simulink model "non_linear_MBS_PF_final"  (R2021b)
settings: Solver=ode15s  StartTime=0  StopTime=SimTime  FixedStep=.00001  RelTol=1e-3
values from neighbouring files (symbols this model uses):
  A_ratio = 1.41    [non_linear_param_MBS_PF_final.m]    (also in non_linear_param_final.m)
  Annulus_area = Bore_area-Rod_area    (= 2356.19449019)    [non_linear_param_MBS_PF_final.m]    (also in non_linear_param_final.m)
  beta_F = 12000e5    (= 1200000000)    [non_linear_param_MBS_PF_final.m]    (also in non_linear_param_final.m)
  Bore_area = pi/4*Bore_diameter^2    (= 3318.30724035)    [non_linear_param_MBS_PF_final.m]    (also in non_linear_param_final.m)
  cyl_c_end = 9e8    (= 900000000)    [non_linear_param_MBS_PF_final.m]    (also in non_linear_param_final.m)
  cyl_c_visc = 1500    [non_linear_param_MBS_PF_final.m]    (also in non_linear_param_final.m)
  cyl_CH_stat = 1/50    (= 0.02)    [non_linear_param_MBS_PF_final.m]    (also in non_linear_param_final.m)
  cyl_F_coul = 75    [non_linear_param_MBS_PF_final.m]    (also in non_linear_param_final.m)
  cyl_FH_stat = 500    [non_linear_param_MBS_PF_final.m]    (also in non_linear_param_final.m)
  cyl_gamma = 250    [non_linear_param_MBS_PF_final.m]    (also in non_linear_param_final.m)
  cyl_k_end = 6e9    (= 6000000000)    [non_linear_param_MBS_PF_final.m]    (also in non_linear_param_final.m)
  cyl_KLi = 0    [non_linear_param_MBS_PF_final.m]    (also in non_linear_param_final.m)
  d_eff_long = sqrt(2*J_sec_long*k_eff_long)    [non_linear_param_MBS_PF_final.m]    (also in non_linear_param_final.m)
  d_eff_short = sqrt(2*J_sec_short*k_eff_short)    [non_linear_param_MBS_PF_final.m]    (also in non_linear_param_final.m)
  DB = 0    [non_linear_param_MBS_PF_final.m]    (also in non_linear_param_final.m = -0.114)
  DR = 1    [non_linear_param_MBS_PF_final.m]    (also in non_linear_param_final.m)
  dr_dcv = 0.7    [non_linear_param_MBS_PF_final.m]    (also in non_linear_param_final.m)
  Eff_Bulk_On = 1    [non_linear_param_MBS_PF_final.m]    (also in non_linear_param_final.m)
  eta_air = 0.7e-2    (= 0.007)    [non_linear_param_MBS_PF_final.m]    (also in non_linear_param_final.m)
  k_cv = 15    [non_linear_param_MBS_PF_final.m]    (also in non_linear_param_final.m)
  k_DCV_PA = 7.85    [non_linear_param_MBS_PF_final.m]    (also in non_linear_param_final.m)
  k_DCV_PB = 7.55    [non_linear_param_MBS_PF_final.m]    (also in non_linear_param_final.m)
  k_DCV_T = 7.85    [non_linear_param_MBS_PF_final.m]    (also in non_linear_param_final.m)
  k_eff_long = E*I/I_sec_long    (= 3050381.94041)    [non_linear_param_MBS_PF_final.m]    (also in non_linear_param_final.m)
  k_eff_short = E*I/I_sec_short    (= 13451432.5427)    [non_linear_param_MBS_PF_final.m]    (also in non_linear_param_final.m)
  k_ocv = 15    [non_linear_param_MBS_PF_final.m]    (also in non_linear_param_final.m)
  K_oil = 1.5e4*0+12000    (= 12000)    [non_linear_param_MBS_PF_final.m]    (also in non_linear_param_final.m)
  k_tanh = 0.005    [non_linear_param_MBS_PF_final.m]    (also in non_linear_param_final.m)
  kappa_air = 1.4    [non_linear_param_MBS_PF_final.m]    (also in non_linear_param_final.m)
  kHP = C*kF_pf_Up    (= 2.90878e-12)    [non_linear_param_MBS_PF_final.m]
  kI = (0.54*ku)/Ti    (= 0.048)    [non_linear_param_MBS_PF_final.m]
  kP = 0.45*ku    (= 0.018)    [non_linear_param_MBS_PF_final.m]
  kq_xS_PB = 4.8946e-04    (= 0.00048946)    [non_linear_param_MBS_PF_final.m]
  KR = 1    [non_linear_param_MBS_PF_final.m]    (also in non_linear_param_final.m)
  mu_ocv = 10    [non_linear_param_MBS_PF_final.m]    (also in non_linear_param_final.m = 3)
  muC = (Bore_diameter^2)/(Bore_diameter^2-Rod_diameter^2)    (= 1.40833333333)    [non_linear_param_MBS_PF_final.m]
  OL_pa = -0.114    [non_linear_param_MBS_PF_final.m]    (also in non_linear_param_final.m)
  OL_pb = 0.114    [non_linear_param_MBS_PF_final.m]    (also in non_linear_param_final.m)
  p0_A = 62.79    [non_linear_param_MBS_PF_final.m]    (also in non_linear_param_final.m)
  p0_A1 = 1.08    [non_linear_param_MBS_PF_final.m]    (also in non_linear_param_final.m)
  p0_B = 1.213    [non_linear_param_MBS_PF_final.m]    (also in non_linear_param_final.m)
  p_cv_fo = 1    [non_linear_param_MBS_PF_final.m]    (also in non_linear_param_final.m)
  p_cv_set = 2.8    [non_linear_param_MBS_PF_final.m]    (also in non_linear_param_final.m)
  p_ocv_fo = 350    [non_linear_param_MBS_PF_final.m]    (also in non_linear_param_final.m)
  p_ocv_set = 196    [non_linear_param_MBS_PF_final.m]    (also in non_linear_param_final.m = 150)
  p_pc_set = 7    [non_linear_param_MBS_PF_final.m]    (also in non_linear_param_final.m)
  p_R = 1.1    [non_linear_param_MBS_PF_final.m]    (also in non_linear_param_final.m)
  p_S = 180    [non_linear_param_MBS_PF_final.m]    (also in non_linear_param_final.m)
  patm = 1.013e5    (= 101300)    [non_linear_param_MBS_PF_final.m]    (also in non_linear_param_final.m)
  Stroke = 500    [non_linear_param_MBS_PF_final.m]    (also in non_linear_param_final.m)
  t0 = 3    [non_linear_param_MBS_PF_final.m]    (also in non_linear_param_final.m = 1)
  tau_ocv = 0.0889*0+0.001    (= 0.001)    [non_linear_param_MBS_PF_final.m]    (also in non_linear_param_final.m)
  tau_pc = tau_ocv    (= 0.001)    [non_linear_param_MBS_PF_final.m]    (also in non_linear_param_final.m)
  tHP = tF_pf_Up/(wn_pf)    (= 0.114613180516)    [non_linear_param_MBS_PF_final.m]
  tStepDown = 4.7*0+7.5    (= 7.5)    [non_linear_param_MBS_PF_final.m]    (also in non_linear_param_final.m = 4.6)
  tStepUp = 10.0    (= 10)    [non_linear_param_MBS_PF_final.m]    (also in non_linear_param_final.m = 6.2)
  tWait = 5.0    (= 5)    [non_linear_param_MBS_PF_final.m]    (also in non_linear_param_final.m = 8)
  UL_at = -0.05    [non_linear_param_MBS_PF_final.m]    (also in non_linear_param_final.m)
  UL_bt = 0.05    [non_linear_param_MBS_PF_final.m]    (also in non_linear_param_final.m)
  Up = 1    [non_linear_param_MBS_PF_final.m]    (also in non_linear_param_final.m)
  uS_sp = -0.4    [non_linear_param_MBS_PF_final.m]    (also in non_linear_param_final.m = -0.55)
  V_CH_A = 1.0e-03    (= 0.001)    [non_linear_param_MBS_PF_final.m]    (also in non_linear_param_final.m)
  V_CH_A1 = 0.1e-03    (= 0.0001)    [non_linear_param_MBS_PF_final.m]    (also in non_linear_param_final.m)
  V_CH_B = 1.25e-03    (= 0.00125)    [non_linear_param_MBS_PF_final.m]    (also in non_linear_param_final.m)
  wn_dcv = 30    [non_linear_param_MBS_PF_final.m]    (also in non_linear_param_final.m)
undefined symbols (not defined in the file or in its folder): sigbuilder_block, smiData, uS0, X, Y, Z
libraries: sm_lib x189, nesl_utility x4, eeGeneralControl x2
(part 6 of 6)

== system non_linear_MBS_PF_final/Test/Subsystem2/Base/Base_profil_base_support_1_RIGID ==
  ReferenceFrame : sm_lib/Frames and Transforms/Reference Frame
  Solid : sm_lib/Body Elements/File Solid  ExtGeomFileName=Base_profil_base_support_Default_sldprt.STEP  UnitType=FromFile  ExtGeomFileUnits=m  StepReaderType=HEX  ExportConvexHullGeometry=off  InertiaType=Custom  Mass=smiData.Solid(3).mass  MassUnits=kg  CenterOfMass=smiData.Solid(3).CoM  CenterOfMassUnits=mm  MomentsOfInertia=smiData.Solid(3).MoI  MomentsOfInertiaUnits=kg*mm^2  ProductsOfInertia=smiData.Solid(3).PoI  ProductsOfInertiaUnits=kg*mm^2  BasedOnType=Density  Density=1000  DensityUnits=kg/m^3  DoExposeReferenceFrame=on  SerializedFrames=<Frames/>  GraphicType=FromGeometry  MarkerShape=Sphere  MarkerSize=10  GraphicVisPropType=SimpleVisualProperties  GraphicDiffuseColor=smiData.Solid(3).color  GraphicSpecularColor=[0.5 0.5 0.5 1.0]  GraphicAmbientColor=[0.15 0.15 0.15 1.0]  GraphicEmissiveColor=[0.0 0.0 0.0 1.0]  GraphicShininess=75  GraphicOpacity=smiData.Solid(3).opacity
  F : PMIOPort  Side=Left
  connections:
  Solid/RConn1 -- ReferenceFrame/RConn1 -- F/RConn1

== system non_linear_MBS_PF_final/Test/Subsystem2/Base/Base_profil_base_support_2_1_RIGID ==
  ReferenceFrame : sm_lib/Frames and Transforms/Reference Frame
  Solid : sm_lib/Body Elements/File Solid  ExtGeomFileName=Base_profil_base_support_2_Default_sldprt.STEP  UnitType=FromFile  ExtGeomFileUnits=m  StepReaderType=HEX  ExportConvexHullGeometry=off  InertiaType=Custom  Mass=smiData.Solid(5).mass  MassUnits=kg  CenterOfMass=smiData.Solid(5).CoM  CenterOfMassUnits=mm  MomentsOfInertia=smiData.Solid(5).MoI  MomentsOfInertiaUnits=kg*mm^2  ProductsOfInertia=smiData.Solid(5).PoI  ProductsOfInertiaUnits=kg*mm^2  BasedOnType=Density  Density=1000  DensityUnits=kg/m^3  DoExposeReferenceFrame=on  SerializedFrames=<Frames/>  GraphicType=FromGeometry  MarkerShape=Sphere  MarkerSize=10  GraphicVisPropType=SimpleVisualProperties  GraphicDiffuseColor=smiData.Solid(5).color  GraphicSpecularColor=[0.5 0.5 0.5 1.0]  GraphicAmbientColor=[0.15 0.15 0.15 1.0]  GraphicEmissiveColor=[0.0 0.0 0.0 1.0]  GraphicShininess=75  GraphicOpacity=smiData.Solid(5).opacity
  F : PMIOPort  Side=Left
  connections:
  Solid/RConn1 -- ReferenceFrame/RConn1 -- F/RConn1

== system non_linear_MBS_PF_final/Test/Subsystem2/Base/Base_profil_cyl_mount1_1_RIGID ==
  ReferenceFrame : sm_lib/Frames and Transforms/Reference Frame
  Solid : sm_lib/Body Elements/File Solid  ExtGeomFileName=Base_profil_cyl_mount1_Default_sldprt.STEP  UnitType=FromFile  ExtGeomFileUnits=m  StepReaderType=HEX  ExportConvexHullGeometry=off  InertiaType=Custom  Mass=smiData.Solid(11).mass  MassUnits=kg  CenterOfMass=smiData.Solid(11).CoM  CenterOfMassUnits=mm  MomentsOfInertia=smiData.Solid(11).MoI  MomentsOfInertiaUnits=kg*mm^2  ProductsOfInertia=smiData.Solid(11).PoI  ProductsOfInertiaUnits=kg*mm^2  BasedOnType=Density  Density=1000  DensityUnits=kg/m^3  DoExposeReferenceFrame=on  SerializedFrames=<Frames/>  GraphicType=FromGeometry  MarkerShape=Sphere  MarkerSize=10  GraphicVisPropType=SimpleVisualProperties  GraphicDiffuseColor=smiData.Solid(11).color  GraphicSpecularColor=[0.5 0.5 0.5 1.0]  GraphicAmbientColor=[0.15 0.15 0.15 1.0]  GraphicEmissiveColor=[0.0 0.0 0.0 1.0]  GraphicShininess=75  GraphicOpacity=smiData.Solid(11).opacity
  F : PMIOPort  Side=Left
  connections:
  Solid/RConn1 -- ReferenceFrame/RConn1 -- F/RConn1

== system non_linear_MBS_PF_final/Test/Subsystem2/Base/Base_profil_cyl_mount_support_sideways_1_RIGID ==
  ReferenceFrame : sm_lib/Frames and Transforms/Reference Frame
  Solid : sm_lib/Body Elements/File Solid  ExtGeomFileName=Base_profil_cyl_mount_support_sideways_Default_sldprt.STEP  UnitType=FromFile  ExtGeomFileUnits=m  StepReaderType=HEX  ExportConvexHullGeometry=off  InertiaType=Custom  Mass=smiData.Solid(2).mass  MassUnits=kg  CenterOfMass=smiData.Solid(2).CoM  CenterOfMassUnits=mm  MomentsOfInertia=smiData.Solid(2).MoI  MomentsOfInertiaUnits=kg*mm^2  ProductsOfInertia=smiData.Solid(2).PoI  ProductsOfInertiaUnits=kg*mm^2  BasedOnType=Density  Density=1000  DensityUnits=kg/m^3  DoExposeReferenceFrame=on  SerializedFrames=<Frames/>  GraphicType=FromGeometry  MarkerShape=Sphere  MarkerSize=10  GraphicVisPropType=SimpleVisualProperties  GraphicDiffuseColor=smiData.Solid(2).color  GraphicSpecularColor=[0.5 0.5 0.5 1.0]  GraphicAmbientColor=[0.15 0.15 0.15 1.0]  GraphicEmissiveColor=[0.0 0.0 0.0 1.0]  GraphicShininess=75  GraphicOpacity=smiData.Solid(2).opacity
  F : PMIOPort  Side=Left
  connections:
  Solid/RConn1 -- ReferenceFrame/RConn1 -- F/RConn1

== system non_linear_MBS_PF_final/Test/Subsystem2/Base/Base_profil_incline_1_RIGID ==
  ReferenceFrame : sm_lib/Frames and Transforms/Reference Frame
  Solid : sm_lib/Body Elements/File Solid  ExtGeomFileName=Base_profil_incline_Default_sldprt.STEP  UnitType=FromFile  ExtGeomFileUnits=m  StepReaderType=HEX  ExportConvexHullGeometry=off  InertiaType=Custom  Mass=smiData.Solid(10).mass  MassUnits=kg  CenterOfMass=smiData.Solid(10).CoM  CenterOfMassUnits=mm  MomentsOfInertia=smiData.Solid(10).MoI  MomentsOfInertiaUnits=kg*mm^2  ProductsOfInertia=smiData.Solid(10).PoI  ProductsOfInertiaUnits=kg*mm^2  BasedOnType=Density  Density=1000  DensityUnits=kg/m^3  DoExposeReferenceFrame=on  SerializedFrames=<Frames/>  GraphicType=FromGeometry  MarkerShape=Sphere  MarkerSize=10  GraphicVisPropType=SimpleVisualProperties  GraphicDiffuseColor=smiData.Solid(10).color  GraphicSpecularColor=[0.5 0.5 0.5 1.0]  GraphicAmbientColor=[0.15 0.15 0.15 1.0]  GraphicEmissiveColor=[0.0 0.0 0.0 1.0]  GraphicShininess=75  GraphicOpacity=smiData.Solid(10).opacity
  F : PMIOPort  Side=Left
  connections:
  Solid/RConn1 -- ReferenceFrame/RConn1 -- F/RConn1

== system non_linear_MBS_PF_final/Test/Subsystem2/Base/Base_profil_incline_2_RIGID ==
  ReferenceFrame : sm_lib/Frames and Transforms/Reference Frame
  Solid : sm_lib/Body Elements/File Solid  ExtGeomFileName=Base_profil_incline_Default_sldprt.STEP  UnitType=FromFile  ExtGeomFileUnits=m  StepReaderType=HEX  ExportConvexHullGeometry=off  InertiaType=Custom  Mass=smiData.Solid(10).mass  MassUnits=kg  CenterOfMass=smiData.Solid(10).CoM  CenterOfMassUnits=mm  MomentsOfInertia=smiData.Solid(10).MoI  MomentsOfInertiaUnits=kg*mm^2  ProductsOfInertia=smiData.Solid(10).PoI  ProductsOfInertiaUnits=kg*mm^2  BasedOnType=Density  Density=1000  DensityUnits=kg/m^3  DoExposeReferenceFrame=on  SerializedFrames=<Frames/>  GraphicType=FromGeometry  MarkerShape=Sphere  MarkerSize=10  GraphicVisPropType=SimpleVisualProperties  GraphicDiffuseColor=smiData.Solid(10).color  GraphicSpecularColor=[0.5 0.5 0.5 1.0]  GraphicAmbientColor=[0.15 0.15 0.15 1.0]  GraphicEmissiveColor=[0.0 0.0 0.0 1.0]  GraphicShininess=75  GraphicOpacity=smiData.Solid(10).opacity
  F : PMIOPort  Side=Left
  connections:
  Solid/RConn1 -- ReferenceFrame/RConn1 -- F/RConn1

== system non_linear_MBS_PF_final/Test/Subsystem2/Base/Base_profil_tower_1_RIGID ==
  ReferenceFrame : sm_lib/Frames and Transforms/Reference Frame
  Solid : sm_lib/Body Elements/File Solid  ExtGeomFileName=Base_profil_tower_Default_sldprt.STEP  UnitType=FromFile  ExtGeomFileUnits=m  StepReaderType=HEX  ExportConvexHullGeometry=off  InertiaType=Custom  Mass=smiData.Solid(8).mass  MassUnits=kg  CenterOfMass=smiData.Solid(8).CoM  CenterOfMassUnits=mm  MomentsOfInertia=smiData.Solid(8).MoI  MomentsOfInertiaUnits=kg*mm^2  ProductsOfInertia=smiData.Solid(8).PoI  ProductsOfInertiaUnits=kg*mm^2  BasedOnType=Density  Density=1000  DensityUnits=kg/m^3  DoExposeReferenceFrame=on  SerializedFrames=<Frames/>  GraphicType=FromGeometry  MarkerShape=Sphere  MarkerSize=10  GraphicVisPropType=SimpleVisualProperties  GraphicDiffuseColor=smiData.Solid(8).color  GraphicSpecularColor=[0.5 0.5 0.5 1.0]  GraphicAmbientColor=[0.15 0.15 0.15 1.0]  GraphicEmissiveColor=[0.0 0.0 0.0 1.0]  GraphicShininess=75  GraphicOpacity=smiData.Solid(8).opacity
  F : PMIOPort  Side=Left
  connections:
  Solid/RConn1 -- ReferenceFrame/RConn1 -- F/RConn1

== system non_linear_MBS_PF_final/Test/Subsystem2/Base/Base_profil_tower_2_RIGID ==
  ReferenceFrame : sm_lib/Frames and Transforms/Reference Frame
  Solid : sm_lib/Body Elements/File Solid  ExtGeomFileName=Base_profil_tower_Default_sldprt.STEP  UnitType=FromFile  ExtGeomFileUnits=m  StepReaderType=HEX  ExportConvexHullGeometry=off  InertiaType=Custom  Mass=smiData.Solid(8).mass  MassUnits=kg  CenterOfMass=smiData.Solid(8).CoM  CenterOfMassUnits=mm  MomentsOfInertia=smiData.Solid(8).MoI  MomentsOfInertiaUnits=kg*mm^2  ProductsOfInertia=smiData.Solid(8).PoI  ProductsOfInertiaUnits=kg*mm^2  BasedOnType=Density  Density=1000  DensityUnits=kg/m^3  DoExposeReferenceFrame=on  SerializedFrames=<Frames/>  GraphicType=FromGeometry  MarkerShape=Sphere  MarkerSize=10  GraphicVisPropType=SimpleVisualProperties  GraphicDiffuseColor=smiData.Solid(8).color  GraphicSpecularColor=[0.5 0.5 0.5 1.0]  GraphicAmbientColor=[0.15 0.15 0.15 1.0]  GraphicEmissiveColor=[0.0 0.0 0.0 1.0]  GraphicShininess=75  GraphicOpacity=smiData.Solid(8).opacity
  F : PMIOPort  Side=Left
  connections:
  Solid/RConn1 -- ReferenceFrame/RConn1 -- F/RConn1

== system non_linear_MBS_PF_final/Test/Subsystem2/Base/Base_profil_tower_3_RIGID ==
  ReferenceFrame : sm_lib/Frames and Transforms/Reference Frame
  Solid : sm_lib/Body Elements/File Solid  ExtGeomFileName=Base_profil_tower_Default_sldprt.STEP  UnitType=FromFile  ExtGeomFileUnits=m  StepReaderType=HEX  ExportConvexHullGeometry=off  InertiaType=Custom  Mass=smiData.Solid(8).mass  MassUnits=kg  CenterOfMass=smiData.Solid(8).CoM  CenterOfMassUnits=mm  MomentsOfInertia=smiData.Solid(8).MoI  MomentsOfInertiaUnits=kg*mm^2  ProductsOfInertia=smiData.Solid(8).PoI  ProductsOfInertiaUnits=kg*mm^2  BasedOnType=Density  Density=1000  DensityUnits=kg/m^3  DoExposeReferenceFrame=on  SerializedFrames=<Frames/>  GraphicType=FromGeometry  MarkerShape=Sphere  MarkerSize=10  GraphicVisPropType=SimpleVisualProperties  GraphicDiffuseColor=smiData.Solid(8).color  GraphicSpecularColor=[0.5 0.5 0.5 1.0]  GraphicAmbientColor=[0.15 0.15 0.15 1.0]  GraphicEmissiveColor=[0.0 0.0 0.0 1.0]  GraphicShininess=75  GraphicOpacity=smiData.Solid(8).opacity
  F : PMIOPort  Side=Left
  connections:
  Solid/RConn1 -- ReferenceFrame/RConn1 -- F/RConn1

== system non_linear_MBS_PF_final/Test/Subsystem2/Base/Base_profil_tower_4_RIGID ==
  ReferenceFrame : sm_lib/Frames and Transforms/Reference Frame
  Solid : sm_lib/Body Elements/File Solid  ExtGeomFileName=Base_profil_tower_Default_sldprt.STEP  UnitType=FromFile  ExtGeomFileUnits=m  StepReaderType=HEX  ExportConvexHullGeometry=off  InertiaType=Custom  Mass=smiData.Solid(8).mass  MassUnits=kg  CenterOfMass=smiData.Solid(8).CoM  CenterOfMassUnits=mm  MomentsOfInertia=smiData.Solid(8).MoI  MomentsOfInertiaUnits=kg*mm^2  ProductsOfInertia=smiData.Solid(8).PoI  ProductsOfInertiaUnits=kg*mm^2  BasedOnType=Density  Density=1000  DensityUnits=kg/m^3  DoExposeReferenceFrame=on  SerializedFrames=<Frames/>  GraphicType=FromGeometry  MarkerShape=Sphere  MarkerSize=10  GraphicVisPropType=SimpleVisualProperties  GraphicDiffuseColor=smiData.Solid(8).color  GraphicSpecularColor=[0.5 0.5 0.5 1.0]  GraphicAmbientColor=[0.15 0.15 0.15 1.0]  GraphicEmissiveColor=[0.0 0.0 0.0 1.0]  GraphicShininess=75  GraphicOpacity=smiData.Solid(8).opacity
  F : PMIOPort  Side=Left
  connections:
  Solid/RConn1 -- ReferenceFrame/RConn1 -- F/RConn1

== system non_linear_MBS_PF_final/Test/Subsystem2/Base/Bearing_house_SKF_SNA_513_611_1_RIGID ==
  ReferenceFrame : sm_lib/Frames and Transforms/Reference Frame
  Solid : sm_lib/Body Elements/File Solid  ExtGeomFileName=Bearing_house_SKF_SNA_513-611_Default_sldprt.STEP  UnitType=FromFile  ExtGeomFileUnits=m  StepReaderType=HEX  ExportConvexHullGeometry=off  InertiaType=Custom  Mass=smiData.Solid(12).mass  MassUnits=kg  CenterOfMass=smiData.Solid(12).CoM  CenterOfMassUnits=mm  MomentsOfInertia=smiData.Solid(12).MoI  MomentsOfInertiaUnits=kg*mm^2  ProductsOfInertia=smiData.Solid(12).PoI  ProductsOfInertiaUnits=kg*mm^2  BasedOnType=Density  Density=1000  DensityUnits=kg/m^3  DoExposeReferenceFrame=on  SerializedFrames=<Frames/>  GraphicType=FromGeometry  MarkerShape=Sphere  MarkerSize=10  GraphicVisPropType=SimpleVisualProperties  GraphicDiffuseColor=smiData.Solid(12).color  GraphicSpecularColor=[0.5 0.5 0.5 1.0]  GraphicAmbientColor=[0.15 0.15 0.15 1.0]  GraphicEmissiveColor=[0.0 0.0 0.0 1.0]  GraphicShininess=75  GraphicOpacity=smiData.Solid(12).opacity
  F : PMIOPort  Side=Left
  connections:
  Solid/RConn1 -- ReferenceFrame/RConn1 -- F/RConn1

== system non_linear_MBS_PF_final/Test/Subsystem2/Base/Bearing_house_SKF_SNA_513_611_2_RIGID ==
  ReferenceFrame : sm_lib/Frames and Transforms/Reference Frame
  Solid : sm_lib/Body Elements/File Solid  ExtGeomFileName=Bearing_house_SKF_SNA_513-611_Default_sldprt.STEP  UnitType=FromFile  ExtGeomFileUnits=m  StepReaderType=HEX  ExportConvexHullGeometry=off  InertiaType=Custom  Mass=smiData.Solid(12).mass  MassUnits=kg  CenterOfMass=smiData.Solid(12).CoM  CenterOfMassUnits=mm  MomentsOfInertia=smiData.Solid(12).MoI  MomentsOfInertiaUnits=kg*mm^2  ProductsOfInertia=smiData.Solid(12).PoI  ProductsOfInertiaUnits=kg*mm^2  BasedOnType=Density  Density=1000  DensityUnits=kg/m^3  DoExposeReferenceFrame=on  SerializedFrames=<Frames/>  GraphicType=FromGeometry  MarkerShape=Sphere  MarkerSize=10  GraphicVisPropType=SimpleVisualProperties  GraphicDiffuseColor=smiData.Solid(12).color  GraphicSpecularColor=[0.5 0.5 0.5 1.0]  GraphicAmbientColor=[0.15 0.15 0.15 1.0]  GraphicEmissiveColor=[0.0 0.0 0.0 1.0]  GraphicShininess=75  GraphicOpacity=smiData.Solid(12).opacity
  F : PMIOPort  Side=Left
  connections:
  Solid/RConn1 -- ReferenceFrame/RConn1 -- F/RConn1

== system non_linear_MBS_PF_final/Test/Subsystem2/Base/Base_bearing_mount_1_RIGID/Base_bearing_mount_sideways_1_RIGID ==
  ReferenceFrame : sm_lib/Frames and Transforms/Reference Frame
  Solid : sm_lib/Body Elements/File Solid  ExtGeomFileName=Base_bearing_mount_sideways_Default_sldprt.STEP  UnitType=FromFile  ExtGeomFileUnits=m  StepReaderType=HEX  ExportConvexHullGeometry=off  InertiaType=Custom  Mass=smiData.Solid(6).mass  MassUnits=kg  CenterOfMass=smiData.Solid(6).CoM  CenterOfMassUnits=mm  MomentsOfInertia=smiData.Solid(6).MoI  MomentsOfInertiaUnits=kg*mm^2  ProductsOfInertia=smiData.Solid(6).PoI  ProductsOfInertiaUnits=kg*mm^2  BasedOnType=Density  Density=1000  DensityUnits=kg/m^3  DoExposeReferenceFrame=on  SerializedFrames=<Frames/>  GraphicType=FromGeometry  MarkerShape=Sphere  MarkerSize=10  GraphicVisPropType=SimpleVisualProperties  GraphicDiffuseColor=smiData.Solid(6).color  GraphicSpecularColor=[0.5 0.5 0.5 1.0]  GraphicAmbientColor=[0.15 0.15 0.15 1.0]  GraphicEmissiveColor=[0.0 0.0 0.0 1.0]  GraphicShininess=75  GraphicOpacity=smiData.Solid(6).opacity
  F : PMIOPort  Side=Left
  connections:
  Solid/RConn1 -- ReferenceFrame/RConn1 -- F/RConn1

== system non_linear_MBS_PF_final/Test/Subsystem2/Base/Base_bearing_mount_1_RIGID/Base_bearing_mount_support_1_RIGID ==
  ReferenceFrame : sm_lib/Frames and Transforms/Reference Frame
  Solid : sm_lib/Body Elements/File Solid  ExtGeomFileName=Base_bearing_mount_support_Default_sldprt.STEP  UnitType=FromFile  ExtGeomFileUnits=m  StepReaderType=HEX  ExportConvexHullGeometry=off  InertiaType=Custom  Mass=smiData.Solid(7).mass  MassUnits=kg  CenterOfMass=smiData.Solid(7).CoM  CenterOfMassUnits=mm  MomentsOfInertia=smiData.Solid(7).MoI  MomentsOfInertiaUnits=kg*mm^2  ProductsOfInertia=smiData.Solid(7).PoI  ProductsOfInertiaUnits=kg*mm^2  BasedOnType=Density  Density=1000  DensityUnits=kg/m^3  DoExposeReferenceFrame=on  SerializedFrames=<Frames/>  GraphicType=FromGeometry  MarkerShape=Sphere  MarkerSize=10  GraphicVisPropType=SimpleVisualProperties  GraphicDiffuseColor=smiData.Solid(7).color  GraphicSpecularColor=[0.5 0.5 0.5 1.0]  GraphicAmbientColor=[0.15 0.15 0.15 1.0]  GraphicEmissiveColor=[0.0 0.0 0.0 1.0]  GraphicShininess=75  GraphicOpacity=smiData.Solid(7).opacity
  F : PMIOPort  Side=Left
  connections:
  Solid/RConn1 -- ReferenceFrame/RConn1 -- F/RConn1

== system non_linear_MBS_PF_final/Test/Subsystem2/Base/Base_bearing_mount_1_RIGID/Base_bearing_mount_support_2_RIGID ==
  ReferenceFrame : sm_lib/Frames and Transforms/Reference Frame
  Solid : sm_lib/Body Elements/File Solid  ExtGeomFileName=Base_bearing_mount_support_Default_sldprt.STEP  UnitType=FromFile  ExtGeomFileUnits=m  StepReaderType=HEX  ExportConvexHullGeometry=off  InertiaType=Custom  Mass=smiData.Solid(7).mass  MassUnits=kg  CenterOfMass=smiData.Solid(7).CoM  CenterOfMassUnits=mm  MomentsOfInertia=smiData.Solid(7).MoI  MomentsOfInertiaUnits=kg*mm^2  ProductsOfInertia=smiData.Solid(7).PoI  ProductsOfInertiaUnits=kg*mm^2  BasedOnType=Density  Density=1000  DensityUnits=kg/m^3  DoExposeReferenceFrame=on  SerializedFrames=<Frames/>  GraphicType=FromGeometry  MarkerShape=Sphere  MarkerSize=10  GraphicVisPropType=SimpleVisualProperties  GraphicDiffuseColor=smiData.Solid(7).color  GraphicSpecularColor=[0.5 0.5 0.5 1.0]  GraphicAmbientColor=[0.15 0.15 0.15 1.0]  GraphicEmissiveColor=[0.0 0.0 0.0 1.0]  GraphicShininess=75  GraphicOpacity=smiData.Solid(7).opacity
  F : PMIOPort  Side=Left
  connections:
  Solid/RConn1 -- ReferenceFrame/RConn1 -- F/RConn1

== matlab function non_linear_MBS_PF_final/Directional Control Valve/Edge Openings/Edge P-A/MATLAB Function1 ==
  data: u_dcv input; u_PA output; OL_pa parameter; OL_pb parameter; UL_at parameter; UL_bt parameter
function u_PA = fcn(u_dcv,OL_pa, OL_pb, UL_at, UL_bt)

  u_PA = 0;

if OL_pa <=  u_dcv 
 u_PA = 0;
 
elseif u_dcv <  OL_pa
  u_PA= -(u_dcv-OL_pa)/(1+OL_pa);
end

== matlab function non_linear_MBS_PF_final/Directional Control Valve/Edge Openings/Edge P-B/MATLAB Function1 ==
  data: u_dcv input; u_PB output; OL_pa parameter; OL_pb parameter; UL_at parameter; UL_bt parameter
function u_PB = fcn(u_dcv,OL_pa, OL_pb, UL_at, UL_bt)

  u_PB = 0;

if u_dcv <=   OL_pb
 u_PB = 0;
 
elseif  OL_pb < u_dcv 
  u_PB = (u_dcv-OL_pb)/(1-OL_pb);
end

== matlab function non_linear_MBS_PF_final/Directional Control Valve/Load-Sensing ==
  data: p_R input; p_B input; p_A1 input; u_dcv input; p_LS output
function p_LS  = fcn(p_R,p_B,p_A1,u_dcv)

p_LS=p_R;

if u_dcv==0
%     if p_A1 > p_B
%     p_LS=p_A1;
%     else
%         p_LS=p_B;
%     end

p_LS = p_R;

end

if u_dcv > 0
    p_LS=p_B;
end

if u_dcv < 0
    p_LS=p_A1;
end
end

== matlab function non_linear_MBS_PF_final/Over-Centre Valve/Capasitance/Bulk Modulus/Effective Bulk Modulus/MATLAB Function ==
  data: p input; K_oil_eff output; patm parameter; eta_air parameter; kappa_air parameter; beta_F parameter
function K_oil_eff = fcn(p,patm, eta_air, kappa_air, beta_F)
p = p*1e5;
% Effective bulk modulus 
beta_eff_Pa  = 1/(1/beta_F+1/(((1-eta_air)*(patm/(p+patm))^(-1/kappa_air)/eta_air+1)*kappa_air*(p+patm)));
K_oil_eff = beta_eff_Pa*1e-5;

== matlab function non_linear_MBS_PF_final/S_shape_Profile/SP_Generator ==
  data: PositionRef output; VelocityRef output; InPositionTime output; s0 input; v0 input; Desired_Position input; VelocitySP input; t0 input; HoldPositionTime input; t input; Error output
function [PositionRef,VelocityRef, InPositionTime, Error] = fcn(s0,v0, Desired_Position,VelocitySP, t0,HoldPositionTime,t)


PositionSP = Desired_Position - s0;

a0 = 0;
j0 = 0;
AccelerationSP = VelocitySP;

vel=VelocitySP;
as=AccelerationSP;
% Input velocity 
vs=vel; %[rad/s]
% Delta velocity 
dv=(vs-v0); %[rad/s]     
% Max Jerk Considerations
jm=as^2/dv; %%[rad/s^3] 
% Constant Velocity periodes
s_acc=(vs^2-v0^2)/as;
CstVelTime=(PositionSP-2*s_acc)/vs;
if CstVelTime < 0
Error = 1;
else 
Error = 0;
end

% Time periodes,
t1=t0+(as/jm);
t2=t1+(as/jm);
t3=t2+CstVelTime;
t4=t3+(as/jm);
t5=t4+(as/jm);
t6=t5+HoldPositionTime;
t7=t6+(as/jm);
t8=t7+(as/jm);
t9=t8+CstVelTime;
t10=t9+(as/jm);
t11=t10+(as/jm);
% Position and Velocity Constants
sh=(v0+(as^2)/(6*jm))*(as/jm);
vh=(vs-v0)/2;
s3=s_acc+vs*(t3-t2);
s4=s3+vs*(t4-t3)-jm*((t4-t3)^3)/6;
v4=vs-jm*((t4-t3)^2)/2;
s5=s0+jm*((t5-t4)^3)/6-as*((t5-t4)^2)/2+v4*(t5-t4)+s4;
%s6=s5+(s0+v0*(t7-t6)+jm*((t7-t6)^3)/6);
s7= s5-(s_acc+vs*(t9-t8));
s8= s7-(vs*(t10-t9)-jm*((t10-t9)^3)/6);
%s9= s8-(jm*((t11-t10)^3)/6-as*((t11-t10)^2)/2+v4*(t11-t10));
%Phase0
if  Error == 1   
s = s0;
v = v0;
a = a0;
j=j0; 
elseif  (t >= 0) && (t <= t0)    
s = s0;
v = v0;
a = a0;
j=j0;        
%Phase1 - concave Acc
elseif  (t >= t0) && (t <= (t1))
s = s0+v0*(t-t0)+jm*((t-t0)^3)/6;
v = v0+jm*((t-t0)^2)/2;
a = jm*(t-t0);
j=jm;
%Phase2 - convex Acc
elseif (t >= (t1)) && (t <= (t2))
s = s0+sh+vh*(t-t1)+as*((t-t1)^2)/2-jm*((t-t1)^3)/6;
v = vh+as*(t-t1)-jm*((t-t1)^2)/2;
a = as-jm*(t-t1);
j = -jm; 
%Phase3 - constant velocity
elseif (t >= t2) && (t <= t3)
s = s0+s_acc+vs*(t-t2);
v = vs;
a = 0;
j = 0;   
%Phase4 - convex Dec
elseif (t >= t3) && (t <= t4)
s = s0+s3+vs*(t-t3)-jm*((t-t3)^3)/6;
v = vs-jm*((t-t3)^2)/2;
a = -jm*(t-t3);
j = -jm; 
%Phase5 - concav Dec
elseif (t >= t4) && (t <= t5)
s = s0+jm*((t-t4)^3)/6-as*((t-t4)^2)/2+v4*(t-t4)+s4;
v = jm*((t-t4)^2)/2-as*(t-t4)+v4;
a = jm*(t-t4)-as;
j=jm; 
%Phase6 - holding position
elseif (t >= t5) && (t <= t6)
s = s5;
v = 0;
a = 0;
j = 0;  
%Phase7 - concave Dec
elseif (t >= t6) && (t <= t7)
s = s5-(v0*(t-t6)+jm*((t-t6)^3)/6);
v = -(v0+jm*((t-t6)^2)/2);
a = -jm*(t-t6);
j=-jm;
%Phase8 - convex Dec
elseif (t >= t7) && (t <= t8)    
s = s5-(sh+vh*(t-t7)+as*((t-t7)^2)/2-jm*((t-t7)^3)/6);
v = -(vh+as*(t-t7)-jm*((t-t7)^2)/2);
a = -(as-jm*(t-t7));
j = jm; 
%Phase9 - constant velocity
elseif (t >= t8) && (t <= t9)
s = s5-(s_acc+vs*(t-t8));
v = -vs;
a = 0;
j = 0;     
%Phase10 - concav Acc
elseif (t >= t9) && (t <= t10)
s = s7-(vs*(t-t9)-jm*((t-t9)^3)/6);
v = -(vs-jm*((t-t9)^2)/2);
a = jm*(t-t9);
j = jm; 
%Phase11 - convex Acc
elseif (t >= t10) && (t <= t11)
s = s8-(jm*((t-t10)^3)/6-as*((t-t10)^2)/2+v4*(t-t10));
v = -(jm*((t-t10)^2)/2-as*(t-t10)+v4);
a = -(jm*(t-t10)-as);
j=-jm; 
%Phase12 - hold position
else
s = s0;
v = v0;
a = a0;
j = j0;
end

if t == 0
PositionRef=s0; %[mm]
else 
PositionRef=s; %[mm]
end 

InPositionTime=t11; %[s]
VelocityRef=v; %[mm/s]
AccelerationRef=a; %[mm/s^2]
JerkRef=j; % %[mm/s^3]

== matlab function non_linear_MBS_PF_final/Subsystem/CH Bore Side/Bulk Modulus/Effective Bulk Modulus/MATLAB Function ==
  data: p input; K_oil_eff output; patm parameter; eta_air parameter; kappa_air parameter; beta_F parameter
function K_oil_eff = fcn(p,patm, eta_air, kappa_air, beta_F)
p = p*1e5;
% Effective bulk modulus 
beta_eff_Pa  = 1/(1/beta_F+1/(((1-eta_air)*(patm/(p+patm))^(-1/kappa_air)/eta_air+1)*kappa_air*(p+patm)));
K_oil_eff = beta_eff_Pa*1e-5;

== matlab function non_linear_MBS_PF_final/Subsystem/CH Rod Side/Bulk Modulus/Effective Bulk Modulus/MATLAB Function ==
  data: p input; K_oil_eff output; patm parameter; eta_air parameter; kappa_air parameter; beta_F parameter
function K_oil_eff = fcn(p,patm, eta_air, kappa_air, beta_F)
p = p*1e5;
% Effective bulk modulus 
beta_eff_Pa  = 1/(1/beta_F+1/(((1-eta_air)*(patm/(p+patm))^(-1/kappa_air)/eta_air+1)*kappa_air*(p+patm)));
K_oil_eff = beta_eff_Pa*1e-5;

== matlab function non_linear_MBS_PF_final/Directional Control Valve/Edge Openings/Edge A-T/MATLAB Function1 ==
  data: u_dcv input; u_AT output; OL_pa parameter; OL_pb parameter; UL_at parameter; UL_bt parameter
function u_AT = fcn(u_dcv,OL_pa, OL_pb, UL_at, UL_bt)

u_AT = 0;

if u_dcv <= UL_at
 u_AT = 0;
 
elseif u_dcv > UL_at   && u_dcv < 2*OL_pb
 u_AT= ((u_dcv-UL_at)*OL_pb)/((1-OL_pb)*(-UL_at+2*OL_pb));
 
elseif 2*OL_pb <= u_dcv
  u_AT= (u_dcv-OL_pb)/(1-OL_pb);
end

== matlab function non_linear_MBS_PF_final/Directional Control Valve/Edge Openings/Edge B-T/MATLAB Function ==
  data: u_dcv input; u_BT output; OL_pa parameter; OL_pb parameter; UL_at parameter; UL_bt parameter
function u_BT = fcn(u_dcv,OL_pa, OL_pb, UL_at, UL_bt)

  u_BT = 0;

if UL_bt <=  u_dcv 
 u_BT = 0;
 
elseif 2*OL_pa < u_dcv && u_dcv < UL_bt
 u_BT= ((u_dcv-UL_bt)*OL_pa)/((1+OL_pa)*(UL_bt-2*OL_pa));
 
elseif u_dcv <=  2*OL_pa
  u_BT= -(u_dcv-OL_pa)/(1+OL_pa);
end

== matlab function non_linear_MBS_PF_final/Anti_Dead_Band ==
  data: DB_Up input; DB_Down input; u_DCV_ref input; u_DCV output
function u_DCV = fcn(DB_Up, DB_Down, u_DCV_ref)



u_DCV = 0;

if (u_DCV_ref < -0.001)  
   u_DCV = -DB_Up + (1-DB_Up)*u_DCV_ref;
elseif (u_DCV_ref > 0.001) 
   u_DCV = DB_Down + (1-DB_Down)*u_DCV_ref;
else
    u_DCV = 0;
end

== matlab function non_linear_MBS_PF_final/Subsystem1/VCC_Step_Function_Validation/StepFunction ==
  data: u_SP input; uS0 input; DB input; t input; t0 input; t1 input; t2 input; y output; T output; t3 input
function [y, T] = step(u_SP, uS0, DB,  t, t0,t1,t2,t3)
y = 0;



if t>=0 && t<t0
    y = uS0;
elseif t>=(t0) && t<(t0+t1)
    y = u_SP+DB; 
elseif t>=(t0+t1) && t<(t0+t1+t2)
    y = uS0; 
elseif t>=(t0+t1+t2) && t<(t0+t1+t2+t3)
    y = -u_SP-DB;          
else
    y = 0; 
end


T = t0+t1+t2+t3;

== matlab function non_linear_MBS_PF_final/Pressure Compensator1/Compensator From Morten Bak Paper ==
  data: p0 input; p_C output; p_S input; p_LS input
function p_C = fcn(p0, p_S, p_LS)

p_C = p_LS;

if p0 <= (p_S-p_LS)
p_C = p_LS+p0;
elseif 0<(p_S-p_LS) < p0
p_C = p_S - p_LS; 
elseif (p_S-p_LS) <= 0
    p_C = p_LS;
end
